simulink model "TosAeb"  (R2021a)
settings: Solver=FixedStepDiscrete  StartTime=0.0  StopTime=inf  FixedStep=0.01  RelTol=1e-3
undefined symbols (not defined in the file or in its folder): ACCModSts, AccSts1, AEB_ObjectMovingOrientation, AEB_ObjectMovingState, CN_BTCActive, CN_CurrentGearR, CN_CurrentGearR_DHT, CN_PTCActive, CN_VDCActive, CN_VSC_EspOff, CN_VSC_FCW_AEB_Error, CN_VSC_FCW_AEB_NoDispay, CN_VSC_FCW_AEB_PerfmDgdtn, CN_VSC_FCW_AEB_TempUnAvlbl, CN_VSC_VehStandstill, CNaccelAvoidMax, CNaccelAvoidMin, CNbrakePedalPress, CNcomfortStrLeftAccelMax, CNcomfortStrLeftAccelMin, CNcomfortStrRightAccelMax, CNcomfortStrRightAccelMin, CNDrvDoorOpen, CNDrvModeEco, CNDrvModeNorm, CNDrvModeOther, CNDrvModeSport, CNDrvSBRreminder, CNNull, CNobjInvalidID, CNPassDoorOpen, CNPassSBRreminder, CNPEBSAccelNegInf, CNPEBSAccelNull, CNPEBSTimeInf, CNPEBSTimeNull, CNSTOPMAX, CNSTOPNULL, CNsubjWidth, CNSwtOFF, CNSwtON, CNTTCMAX, CNTTCNULL, CNVehConfigAEBCycNone, CNVehConfigAEBNone, CNVehConfigAEBPedNone, CNVehConfigFCWNone, CNVehConfigJANone, CollisionSeverity, FALSE, FCWSnvty, JACycObjCalcInfoDefaultParams, JAPedObjCalcInfoDefaultParams, JAVehObjCalcInfoDefaultParams, latCycObjCalcInfoDefaultParams, latPedObjCalcInfoDefaultParams, longObjCalcInfoDefaultParams, ModSts, movingStatus, ObjectType ... (+1112)
libraries: simulink x3031, AEBSFunctionLib x50, Ta_Library x33, AEBSStateMachineLib x23, Mylibrary x12, simulink_extras x7, ObjSelect_lib x3, dspstat3 x2
(part 41 of 102)

== system TosAeb/FcwAebJa_Step_sys/ALG_AEBSystem/ALG_AEBController/AEB_FuncCondCalcApp/LongFunctionCondCalc/vehAEBpartSuppAborCondCalc/vehAEBpartAborCondCalc/vehAEBpartSituationRelAborCondCalc ==
  overlapRatio : Inport
  dxFrontBumper : Inport  Port=2
  probEscape : Inport  Port=3
  Compare To Constant3 : simulink/Logic and Bit Operations/Compare To Constant  relop=>  const=P_AEB_Func_vehAEBpartEscapeAborThres  OutDataTypeStr=boolean
  Compare To Constant6 : simulink/Logic and Bit Operations/Compare To Constant  relop===  const=FALSE  OutDataTypeStr=boolean
  Logical Operator1 : Logic  Operator=OR  AllPortsSameDT=off  OutDataTypeStr=boolean
  OverlapThesLookupTable : Lookup_n-D  NumberOfTableDimensions=1  BreakpointsForDimension1=P_AEB_Func_vehAEBpartOverlapAborThresX  BeginIndexSearchUsingPreviousIndexResult=on  Table=P_AEB_Func_vehAEBpartOverlapAborThresY  ExtrapMethod=Clip  InternalRulePriority=Speed  RndMeth=Simplest  InputSameDT=on
  Relational Operator : RelationalOperator  Operator=>  OutDataTypeStr=boolean  RndMeth=Simplest
  vehAEBpartSituationRelAborCondFulfilled : Outport
  wires:
  probEscape/1 -> Compare To Constant3/1
  dxFrontBumper/1 -> OverlapThesLookupTable/1
  OverlapThesLookupTable/1 -> Relational Operator/2
  Relational Operator/1 -> Compare To Constant6/1  "OverlapCondFulfilled"
  Compare To Constant3/1 -> Logical Operator1/2  "probEscapeFulfilled"
  overlapRatio/1 -> Relational Operator/1
  Logical Operator1/1 -> vehAEBpartSituationRelAborCondFulfilled/1
  Compare To Constant6/1 -> Logical Operator1/1  "OverlapCondFulfilled"

== system TosAeb/FcwAebJa_Step_sys/ALG_AEBSystem/ALG_AEBController/AEB_FuncCondCalcApp/LongFunctionCondCalc/vehAEBpartSuppAborCondCalc/vehAEBpartAborCondCalc/vehAEBpartVehRelAborCondCalc ==
  SteerWheelAngle : Inport
  SteerWheelSpd : Inport  Port=2
  AccrPedlPosnDiagc : Inport  Port=3
  vehStateCalcAEBpartAborFlg : Inport  Port=4
  DriverAborCondJudge : Subsystem
  vehPreWarningVehRelActiveCondJudge : Subsystem
  vehAEBpartVehRelAborCondFulfilled : Outport
  wires:
  vehStateCalcAEBpartAborFlg/1 -> vehPreWarningVehRelActiveCondJudge/2(vehStateCalcAEBpartAborFlg)
  AccrPedlPosnDiagc/1 -> DriverAborCondJudge/3(AccrPedlPosnDiagc)
  SteerWheelSpd/1 -> DriverAborCondJudge/2(SteerWheelSpd)
  SteerWheelAngle/1 -> DriverAborCondJudge/1(SteerWheelAngle)
  DriverAborCondJudge/1(driverAborCondFlg) -> vehPreWarningVehRelActiveCondJudge/1(driverAborCondFlg)
  vehPreWarningVehRelActiveCondJudge/1(vehAEBpartVehRelAborCondFulfilled) -> vehAEBpartVehRelAborCondFulfilled/1

== system TosAeb/FcwAebJa_Step_sys/ALG_AEBSystem/ALG_AEBController/AEB_FuncCondCalcApp/LongFunctionCondCalc/vehAEBpartSuppAborCondCalc/vehAEBpartSuppCondCalc/vehAEBpartObjRelActiveCondCalc ==
  ObjectMovingState : Inport
  ObjectTypeCameraConfirmed : Inport  Port=2
  ObjectType : Inport  Port=3
  subjCurvTraj : Inport  Port=4
  subjLatAccel : Inport  Port=5
  subjVel : Inport  Port=6
  vehConfigAEBCyc : Inport  Port=7
  vehConfigAEBPed : Inport  Port=8
  AEB_PedResp : Inport  Port=9
  ObjectMovingOrientation : Inport  Port=10
  ObjectID : Inport  Port=11
  ObjectExistProb : Inport  Port=12
  ObjectIsObstacleProb : Inport  Port=13
  dxVariance : Inport  Port=14
  dxFrontBumper : Inport  Port=15
  ObjFusQuality : Inport  Port=16
  AEBpartVruFuncConfig : Subsystem
  Compare To Constant1 : simulink/Logic and Bit Operations/Compare To Constant  relop===  const=AEB_ObjectMovingState.Moving  OutDataTypeStr=boolean
  Compare To Constant10 : simulink/Logic and Bit Operations/Compare To Constant  relop=<  const=P_AEB_Func_vehAEBpartDxActivationThres  OutDataTypeStr=boolean
  Compare To Constant11 : simulink/Logic and Bit Operations/Compare To Constant  relop===  const=TRUE  OutDataTypeStr=boolean
  Compare To Constant12 : simulink/Logic and Bit Operations/Compare To Constant  relop===  const=FALSE  OutDataTypeStr=boolean
  Compare To Constant2 : simulink/Logic and Bit Operations/Compare To Constant  relop===  const=AEB_ObjectMovingState.Stopped  OutDataTypeStr=boolean
  Compare To Constant3 : simulink/Logic and Bit Operations/Compare To Constant  relop===  const=AEB_ObjectMovingOrientation.SameDir  OutDataTypeStr=boolean
  Compare To Constant4 : simulink/Logic and Bit Operations/Compare To Constant  relop=~=  const=CNobjInvalidID  OutDataTypeStr=boolean
  Compare To Constant5 : simulink/Logic and Bit Operations/Compare To Constant  relop=>  const=P_AEB_Func_vehAEBpartObjObstacleProbActivationThres  OutDataTypeStr=boolean
  Compare To Constant6 : simulink/Logic and Bit Operations/Compare To Constant  relop=~=  const=CNobjInvalidID  OutDataTypeStr=boolean
  Compare To Constant7 : simulink/Logic and Bit Operations/Compare To Constant  relop===  const=TRUE  OutDataTypeStr=boolean
  Compare To Constant8 : simulink/Logic and Bit Operations/Compare To Constant  relop===  const=TRUE  OutDataTypeStr=boolean
  Compare To Constant9 : simulink/Logic and Bit Operations/Compare To Constant  relop=<  const=P_AEB_Func_vehAEBpartDxVarActivationThres  OutDataTypeStr=boolean
  Constant1 : Constant  Value=P_AEB_Func_vehAEBpartSubjVelActivationThresForPedCyc
  Constant2 : Constant  Value=P_AEB_Func_vehAEBpartSubjCurvTrajActivationThresForPedCyc
  Constant3 : Constant  Value=P_AEB_Func_vehAEBpartSubjLatAccelActivationThresForPedCyc
  Logical Operator : Logic  Operator=OR  AllPortsSameDT=off  OutDataTypeStr=boolean
  Logical Operator1 : Logic  AllPortsSameDT=off  OutDataTypeStr=boolean
  Logical Operator2 : Logic  AllPortsSameDT=off  OutDataTypeStr=boolean
  Logical Operator5 : Logic  Inputs=10  AllPortsSameDT=off  OutDataTypeStr=boolean
  PedCycObjCondSuppJudgeFlow : AEBSFunctionLib/PedCycObjCondSuppJudgeFlow  SystemSampleTime=-1  VariantActivationTime=update diagram  Latency=0  AutoFrameSizeCalculation=off
  Product : Product
  Relational Operator : RelationalOperator  Operator===  OutDataTypeStr=boolean  RndMeth=Simplest
  Unit Delay : UnitDelay  SampleTime=-1  HasFrameUpgradeWarning=on
  vehAEBpartObjRelActiveCondFulfilled : Outport
  wires:
  Compare To Constant11/1 -> Logical Operator5/4  "vehAEBpartVruFuncConfig"
  AEBpartVruFuncConfig/1(vehAEBpartVruFuncConfig) -> Compare To Constant11/1
  Compare To Constant7/1 -> Logical Operator5/10  "ObjFusQuality"
  ObjFusQuality/1 -> Compare To Constant7/1
  ObjectMovingState/1 -> Compare To Constant2/1, Compare To Constant1/1
  Compare To Constant8/1 -> Logical Operator5/2  "ObjFusionCamerainfoConfm"
  Constant3/1 -> PedCycObjCondSuppJudgeFlow/11
  Constant2/1 -> PedCycObjCondSuppJudgeFlow/10
  Constant1/1 -> PedCycObjCondSuppJudgeFlow/9
  AEB_PedResp/1 -> PedCycObjCondSuppJudgeFlow/8
  vehConfigAEBPed/1 -> PedCycObjCondSuppJudgeFlow/7
  vehConfigAEBCyc/1 -> PedCycObjCondSuppJudgeFlow/6
  subjVel/1 -> PedCycObjCondSuppJudgeFlow/5
  subjLatAccel/1 -> PedCycObjCondSuppJudgeFlow/4
  subjCurvTraj/1 -> PedCycObjCondSuppJudgeFlow/3
  ObjectType/1 -> PedCycObjCondSuppJudgeFlow/2, AEBpartVruFuncConfig/1(ObjectType)
  ObjectTypeCameraConfirmed/1 -> PedCycObjCondSuppJudgeFlow/1, Compare To Constant8/1
  Compare To Constant9/1 -> Logical Operator5/8  "dxRelVarCondFulfilled"
  Logical Operator1/1 -> Logical Operator5/6  "objIDCondFulfilled"
  Logical Operator/1 -> Logical Operator5/1  "objMovingStateCondFulfilled"
  Compare To Constant10/1 -> Logical Operator5/9  "dxRelCondFulfilled"
  PedCycObjCondSuppJudgeFlow/1 -> Compare To Constant12/1  "pedCycObjCondSuppFlg"
  Logical Operator2/1 -> Logical Operator5/7  "objQualityCondFulfilled"
  Compare To Constant3/1 -> Logical Operator5/5  "objMovingOrientationCondFulfilled"
  Logical Operator5/1 -> vehAEBpartObjRelActiveCondFulfilled/1  "vehAEBpartObjRelActiveCondFulfilled"
  Compare To Constant4/1 -> Logical Operator1/1
  Relational Operator/1 -> Logical Operator1/2
  dxVariance/1 -> Compare To Constant9/1
  dxFrontBumper/1 -> Compare To Constant10/1
  Product/1 -> Compare To Constant5/1
  Compare To Constant5/1 -> Logical Operator2/2
  Compare To Constant6/1 -> Logical Operator2/1
  ObjectIsObstacleProb/1 -> Product/2
  ObjectExistProb/1 -> Product/1
  Unit Delay/1 -> Relational Operator/2
  ObjectID/1 -> Compare To Constant4/1, Relational Operator/1, Unit Delay/1, Compare To Constant6/1
  ObjectMovingOrientation/1 -> Compare To Constant3/1
  Compare To Constant1/1 -> Logical Operator/1
  Compare To Constant2/1 -> Logical Operator/2
  Compare To Constant12/1 -> Logical Operator5/3  "pedCycReleventCondFulfilled"

== system TosAeb/FcwAebJa_Step_sys/ALG_AEBSystem/ALG_AEBController/AEB_FuncCondCalcApp/LongFunctionCondCalc/vehAEBpartSuppAborCondCalc/vehAEBpartSuppCondCalc/vehAEBpartSituationRelActiveCondCalc ==
  ObjectTypeCameraConfirmed : Inport
  dxFrontBumper : Inport  Port=2
  longCollisionSeverity : Inport  Port=3
  overlapRatio : Inport  Port=4
  LCK_Active : Inport  Port=5
  probEscape : Inport  Port=6
  subjCurvTraj : Inport  Port=7
  overlapThesCalc : Subsystem
  vehAEBpartSituationRelActiveCondCalc : Subsystem
  vehAEBpartSituationRelActiveCondFulfilled : Outport
  wires:
  LCK_Active/1 -> vehAEBpartSituationRelActiveCondCalc/4(LCK_Active)
  vehAEBpartSituationRelActiveCondCalc/1(vehAEBpartSituationRelActiveCondFulfilled) -> vehAEBpartSituationRelActiveCondFulfilled/1  "vehAEBpartSituationRelActiveCondFulfilled"
  subjCurvTraj/1 -> vehAEBpartSituationRelActiveCondCalc/6(subjCurvTraj)
  probEscape/1 -> vehAEBpartSituationRelActiveCondCalc/5(probEscape)
  overlapThesCalc/1(overlapThes) -> vehAEBpartSituationRelActiveCondCalc/3(OverlapThes)
  overlapRatio/1 -> vehAEBpartSituationRelActiveCondCalc/2(overlapRatio)
  longCollisionSeverity/1 -> vehAEBpartSituationRelActiveCondCalc/1(longCollisionSeverity)
  ObjectTypeCameraConfirmed/1 -> overlapThesCalc/1(ObjectTypeCameraConfirmed)
  dxFrontBumper/1 -> overlapThesCalc/2(dxFrontBumper)

== system TosAeb/FcwAebJa_Step_sys/ALG_AEBSystem/ALG_AEBController/AEB_FuncCondCalcApp/LongFunctionCondCalc/vehAEBpartSuppAborCondCalc/vehAEBpartSuppCondCalc/vehAEBpartSuppCondFinalJudge ==
  vehAEBpartObjRelActiveCondFulfilled : Inport
  vehAEBpartVehRelActiveCondFulfilled : Inport  Port=2
  vehAEBpartSituationRelActiveCondFulfilled : Inport  Port=3
  Compare To Constant : simulink/Logic and Bit Operations/Compare To Constant  relop===  const=FALSE  OutDataTypeStr=boolean
  Compare To Constant1 : simulink/Logic and Bit Operations/Compare To Constant  relop===  const=FALSE  OutDataTypeStr=boolean
  Compare To Constant2 : simulink/Logic and Bit Operations/Compare To Constant  relop===  const=FALSE  OutDataTypeStr=boolean
  Logical Operator : Logic  Operator=OR  Inputs=3  AllPortsSameDT=off  OutDataTypeStr=boolean
  vehAEBpartSuppCondFulfilled : Outport
  wires:
  Compare To Constant2/1 -> Logical Operator/3
  Compare To Constant1/1 -> Logical Operator/2
  Compare To Constant/1 -> Logical Operator/1
  Logical Operator/1 -> vehAEBpartSuppCondFulfilled/1  "vehAEBpartSuppCondFulfilled"
  vehAEBpartSituationRelActiveCondFulfilled/1 -> Compare To Constant2/1  "vehAEBpartSituationRelActiveCondFulfilled"
  vehAEBpartObjRelActiveCondFulfilled/1 -> Compare To Constant/1  "vehAEBpartObjRelActiveCondFulfilled"
  vehAEBpartVehRelActiveCondFulfilled/1 -> Compare To Constant1/1  "vehAEBpartVehRelActiveCondFulfilled"

== system TosAeb/FcwAebJa_Step_sys/ALG_AEBSystem/ALG_AEBController/AEB_FuncCondCalcApp/LongFunctionCondCalc/vehAEBpartSuppAborCondCalc/vehAEBpartSuppCondCalc/vehAEBpartVehRelActiveCondCalc ==
  subjVel : Inport
  SteerWheelAngle : Inport  Port=2
  SteerWheelSpd : Inport  Port=3
  AccrPedlPosnDiagc : Inport  Port=4
  VehConfigIbooster : Inport  Port=5
  ESPmasterCylindBrakePress : Inport  Port=6
  BrakePedalStatus_G : Inport  Port=7
  vehStateCalcAEBpartSuppFlg : Inport  Port=8
  IB_BrkPedalAppld : Inport  Port=9
  DriverCondJudge : Subsystem
  vehAEBpartVehRelActiveCondJudge : Subsystem
  vehAEBpartVehRelActiveCondFulfilled : Outport
  wires:
  VehConfigIbooster/1 -> DriverCondJudge/4(VehConfigIbooster)
  IB_BrkPedalAppld/1 -> DriverCondJudge/7(IB_BrkPedalAppld)
  vehAEBpartVehRelActiveCondJudge/1(vehAEBpartVehRelActiveCondFulfilled) -> vehAEBpartVehRelActiveCondFulfilled/1  "vehAEBpartVehRelActiveCondFulfilled"
  vehStateCalcAEBpartSuppFlg/1 -> vehAEBpartVehRelActiveCondJudge/3(vehStateCalcAEBpartSuppFlg)
  subjVel/1 -> vehAEBpartVehRelActiveCondJudge/1(subjVel)
  DriverCondJudge/1(driverSuppCondFlg) -> vehAEBpartVehRelActiveCondJudge/2(driverSuppCondFlg)
  AccrPedlPosnDiagc/1 -> DriverCondJudge/3(AccrPedlPosnDiagc)
  ESPmasterCylindBrakePress/1 -> DriverCondJudge/5(ESPmasterCylindBrakePress)
  SteerWheelSpd/1 -> DriverCondJudge/2(SteerWheelSpd)
  SteerWheelAngle/1 -> DriverCondJudge/1(SteerWheelAngle)
  BrakePedalStatus_G/1 -> DriverCondJudge/6(BrakePedalStatus_G)

== system TosAeb/FcwAebJa_Step_sys/ALG_AEBSystem/ALG_AEBController/AEB_FuncCondCalcApp/LongFunctionCondCalc/vehAcuteWarningSuppAborCondFulfilled/vehAcuteWarningAborCondCalc/vehAcuteWarningAborCondFinalJudge ==
  vehAcuteWarningObjRelAborCondFulfilled : Inport
  vehAcuteWarningVehRelAborCondFulfilled : Inport  Port=2
  vehAcuteWarningSituationRelAborCondFulfilled : Inport  Port=3
  Compare To Constant1 : simulink/Logic and Bit Operations/Compare To Constant  relop===  const=TRUE  OutDataTypeStr=boolean
  Compare To Constant2 : simulink/Logic and Bit Operations/Compare To Constant  relop===  const=TRUE  OutDataTypeStr=boolean
  Compare To Constant3 : simulink/Logic and Bit Operations/Compare To Constant  relop===  const=TRUE  OutDataTypeStr=boolean
  Logical Operator : Logic  Operator=OR  Inputs=3  AllPortsSameDT=off  OutDataTypeStr=boolean
  vehAcuteWarningAborCond : Outport
  wires:
  Compare To Constant3/1 -> Logical Operator/3
  Compare To Constant2/1 -> Logical Operator/2
  Compare To Constant1/1 -> Logical Operator/1
  vehAcuteWarningVehRelAborCondFulfilled/1 -> Compare To Constant2/1
  vehAcuteWarningObjRelAborCondFulfilled/1 -> Compare To Constant1/1
  vehAcuteWarningSituationRelAborCondFulfilled/1 -> Compare To Constant3/1
  Logical Operator/1 -> vehAcuteWarningAborCond/1  "vehAcuteWarningAborCond"

== system TosAeb/FcwAebJa_Step_sys/ALG_AEBSystem/ALG_AEBController/AEB_FuncCondCalcApp/LongFunctionCondCalc/vehAcuteWarningSuppAborCondFulfilled/vehAcuteWarningAborCondCalc/vehAcuteWarningObjRelAborCondCalc ==
  ObjectID : Inport
  Compare To Constant4 : simulink/Logic and Bit Operations/Compare To Constant  relop===  const=CNobjInvalidID  OutDataTypeStr=boolean
  Logical Operator1 : Logic  Operator=OR  AllPortsSameDT=off  OutDataTypeStr=boolean
  Relational Operator : RelationalOperator  Operator=~=  OutDataTypeStr=boolean  RndMeth=Simplest
  Unit Delay : UnitDelay  SampleTime=-1  HasFrameUpgradeWarning=on
  vehAcuteWarningObjRelAborCondFulfilled : Outport
  wires:
  Logical Operator1/1 -> vehAcuteWarningObjRelAborCondFulfilled/1  "vehAcuteWarningObjRelAborCondFulfilled"
  Compare To Constant4/1 -> Logical Operator1/1
  Relational Operator/1 -> Logical Operator1/2
  Unit Delay/1 -> Relational Operator/2
  ObjectID/1 -> Compare To Constant4/1, Relational Operator/1, Unit Delay/1

== system TosAeb/FcwAebJa_Step_sys/ALG_AEBSystem/ALG_AEBController/AEB_FuncCondCalcApp/LongFunctionCondCalc/vehAcuteWarningSuppAborCondFulfilled/vehAcuteWarningAborCondCalc/vehAcuteWarningSituationRelAborCondCalc ==
  overlapRatio : Inport
  dxFrontBumper : Inport  Port=2
  probEscape : Inport  Port=3
  Compare To Constant3 : simulink/Logic and Bit Operations/Compare To Constant  relop=>  const=P_AEB_Func_vehAcuteWarningEscapeAborThres  OutDataTypeStr=boolean
  Compare To Constant6 : simulink/Logic and Bit Operations/Compare To Constant  relop===  const=FALSE  OutDataTypeStr=boolean
  Logical Operator1 : Logic  AllPortsSameDT=off  OutDataTypeStr=boolean
  OverlapThesLookupTable : Lookup_n-D  NumberOfTableDimensions=1  BreakpointsForDimension1=P_AEB_Func_vehAcuteWarningOverlapAborThresX  BeginIndexSearchUsingPreviousIndexResult=on  Table=P_AEB_Func_vehAcuteWarningOverlapAborThresY  ExtrapMethod=Clip  InternalRulePriority=Speed  RndMeth=Simplest  InputSameDT=on
  Relational Operator : RelationalOperator  Operator=>  OutDataTypeStr=boolean  RndMeth=Simplest
  vehAcuteWarningSituationRelAborCondFulfilled : Outport
  wires:
  Compare To Constant6/1 -> Logical Operator1/1
  Logical Operator1/1 -> vehAcuteWarningSituationRelAborCondFulfilled/1  "vehAcuteWarningSituationRelABorCondFulfilled"
  overlapRatio/1 -> Relational Operator/1
  Compare To Constant3/1 -> Logical Operator1/2  "probEscapeFulfilled"
  Relational Operator/1 -> Compare To Constant6/1  "OverlapCondFulfilled"
  OverlapThesLookupTable/1 -> Relational Operator/2
  dxFrontBumper/1 -> OverlapThesLookupTable/1
  probEscape/1 -> Compare To Constant3/1

== system TosAeb/FcwAebJa_Step_sys/ALG_AEBSystem/ALG_AEBController/AEB_FuncCondCalcApp/LongFunctionCondCalc/vehAcuteWarningSuppAborCondFulfilled/vehAcuteWarningAborCondCalc/vehAcuteWarningVehRelAborCondCalc ==
  SteerWheelAngle : Inport
  SteerWheelSpd : Inport  Port=2
  ESPmasterCylindBrakePress : Inport  Port=3
  vehStateCalcAcuteWarningAborFlg : Inport  Port=4
  DriverAborCondJudge : Subsystem
  vehPreWarningVehRelActiveCondJudge : Subsystem
  vehAcuteWarningVehRelAborCondFulfilled : Outport
  wires:
  vehPreWarningVehRelActiveCondJudge/1(vehAcuteWarningVehRelAborCondFulfilled) -> vehAcuteWarningVehRelAborCondFulfilled/1  "vehAcuteWarningVehRelAborCondFulfilled"
  vehStateCalcAcuteWarningAborFlg/1 -> vehPreWarningVehRelActiveCondJudge/2(vehStateCalcAcuteWarningAborFlg)
  DriverAborCondJudge/1(driverAborCondFlg) -> vehPreWarningVehRelActiveCondJudge/1(driverAborCondFlg)
  ESPmasterCylindBrakePress/1 -> DriverAborCondJudge/3(AccrPedlPosnDiagc)
  SteerWheelSpd/1 -> DriverAborCondJudge/2(SteerWheelSpd)
  SteerWheelAngle/1 -> DriverAborCondJudge/1(SteerWheelAngle)

== system TosAeb/FcwAebJa_Step_sys/ALG_AEBSystem/ALG_AEBController/AEB_FuncCondCalcApp/LongFunctionCondCalc/vehAcuteWarningSuppAborCondFulfilled/vehAcuteWarningSuppCondCalc/vehAcuteWarningObjRelActiveCondCalc ==
  ObjectMovingState : Inport
  dz : Inport  Port=2
  ObjectTypeCameraConfirmed : Inport  Port=3
  ObjectType : Inport  Port=4
  subjCurvTraj : Inport  Port=5
  subjLatAccel : Inport  Port=6
  subjVel : Inport  Port=7
  vehConfigAEBCyc : Inport  Port=8
  vehConfigAEBPed : Inport  Port=9
  AEB_PedResp : Inport  Port=10
  ObjectMovingOrientation : Inport  Port=11
  ObjectID : Inport  Port=12
  ObjectExistProb : Inport  Port=13
  ObjectIsObstacleProb : Inport  Port=14
  dxVariance : Inport  Port=15
  dxFrontBumper : Inport  Port=16
  ObjFusQuality : Inport  Port=17
  Compare To Constant : simulink/Logic and Bit Operations/Compare To Constant  relop===  const=AEB_ObjectMovingState.Still  OutDataTypeStr=boolean
  Compare To Constant1 : simulink/Logic and Bit Operations/Compare To Constant  relop===  const=AEB_ObjectMovingState.Moving  OutDataTypeStr=boolean
  Compare To Constant10 : simulink/Logic and Bit Operations/Compare To Constant  relop=<  const=P_AEB_Func_vehAcuteWarningDxActivationThres  OutDataTypeStr=boolean
  Compare To Constant11 : simulink/Logic and Bit Operations/Compare To Constant  relop===  const=TRUE  OutDataTypeStr=boolean
  Compare To Constant12 : simulink/Logic and Bit Operations/Compare To Constant  relop===  const=FALSE  OutDataTypeStr=boolean
  Compare To Constant14 : simulink/Logic and Bit Operations/Compare To Constant  relop===  const=AEB_ObjectMovingState.Still  OutDataTypeStr=boolean
  Compare To Constant2 : simulink/Logic and Bit Operations/Compare To Constant  relop===  const=AEB_ObjectMovingState.Stopped  OutDataTypeStr=boolean
  Compare To Constant3 : simulink/Logic and Bit Operations/Compare To Constant  relop===  const=AEB_ObjectMovingOrientation.SameDir  OutDataTypeStr=boolean
  Compare To Constant4 : simulink/Logic and Bit Operations/Compare To Constant  relop=~=  const=CNobjInvalidID  OutDataTypeStr=boolean
  Compare To Constant5 : simulink/Logic and Bit Operations/Compare To Constant  relop=>  const=P_AEB_Func_vehAcuteWarningObjObstacleProbActivationThres  OutDataTypeStr=boolean
  Compare To Constant6 : simulink/Logic and Bit Operations/Compare To Constant  relop=~=  const=CNobjInvalidID  OutDataTypeStr=boolean
  Compare To Constant7 : simulink/Logic and Bit Operations/Compare To Constant  relop=<  const=P_AEB_Func_vehAcuteWarningObjHeightActivationThres  OutDataTypeStr=boolean
  Compare To Constant8 : simulink/Logic and Bit Operations/Compare To Constant  relop===  const=TRUE  OutDataTypeStr=boolean
  Compare To Constant9 : simulink/Logic and Bit Operations/Compare To Constant  relop=<  const=P_AEB_Func_vehAcuteWarningDxVarActivationThres  OutDataTypeStr=boolean
  Constant : Constant  Value=TRUE
  Constant1 : Constant  Value=P_AEB_Func_vehAcuteWarningSubjVelActivationThresForPedCyc
  Constant2 : Constant  Value=P_AEB_Func_vehAcuteWarningSubjCurvTrajActivationThresForPedCyc
  Constant3 : Constant  Value=P_AEB_Func_vehAcuteWarningSubjLatAccelActivationThresForPedCyc
  Logical Operator : Logic  Operator=OR  Inputs=3  AllPortsSameDT=off  OutDataTypeStr=boolean
  Logical Operator1 : Logic  AllPortsSameDT=off  OutDataTypeStr=boolean
  Logical Operator2 : Logic  AllPortsSameDT=off  OutDataTypeStr=boolean
  Logical Operator5 : Logic  Inputs=10  AllPortsSameDT=off  OutDataTypeStr=boolean
  PedCycObjCondSuppJudgeFlow : AEBSFunctionLib/PedCycObjCondSuppJudgeFlow  SystemSampleTime=-1  VariantActivationTime=update diagram  Latency=0  AutoFrameSizeCalculation=off
  Product : Product
  Relational Operator : RelationalOperator  Operator===  OutDataTypeStr=boolean  RndMeth=Simplest
  Switch : Switch  Criteria=u2 ~= 0
  Unit Delay : UnitDelay  SampleTime=-1  HasFrameUpgradeWarning=on
  vehAcuteWarningObjRelActiveCondFulfilled : Outport
  wires:
  Compare To Constant11/1 -> Logical Operator5/10  "ObjFusQuality"
  ObjFusQuality/1 -> Compare To Constant11/1
  Compare To Constant8/1 -> Logical Operator5/3  "objCameraConfmFullfilled"
  Constant3/1 -> PedCycObjCondSuppJudgeFlow/11
  Constant2/1 -> PedCycObjCondSuppJudgeFlow/10
  Constant1/1 -> PedCycObjCondSuppJudgeFlow/9
  subjCurvTraj/1 -> PedCycObjCondSuppJudgeFlow/3
  subjLatAccel/1 -> PedCycObjCondSuppJudgeFlow/4
  subjVel/1 -> PedCycObjCondSuppJudgeFlow/5
  vehConfigAEBCyc/1 -> PedCycObjCondSuppJudgeFlow/6
  vehConfigAEBPed/1 -> PedCycObjCondSuppJudgeFlow/7
  AEB_PedResp/1 -> PedCycObjCondSuppJudgeFlow/8
  ObjectType/1 -> PedCycObjCondSuppJudgeFlow/2
  ObjectTypeCameraConfirmed/1 -> PedCycObjCondSuppJudgeFlow/1, Compare To Constant8/1
  Constant/1 -> Switch/3
  Compare To Constant7/1 -> Switch/1
  ObjectMovingState/1 -> Compare To Constant14/1, Compare To Constant/1, Compare To Constant2/1, Compare To Constant1/1
  Compare To Constant14/1 -> Switch/2
  Compare To Constant12/1 -> Logical Operator5/4  "pedCycReleventCondFulfilled"
  Switch/1 -> Logical Operator5/2  "objHeightInfoFulfilled"
  Compare To Constant2/1 -> Logical Operator/2
  Compare To Constant1/1 -> Logical Operator/1
  ObjectMovingOrientation/1 -> Compare To Constant3/1
  ObjectID/1 -> Compare To Constant6/1, Unit Delay/1, Relational Operator/1, Compare To Constant4/1
  Unit Delay/1 -> Relational Operator/2
  ObjectExistProb/1 -> Product/1
  ObjectIsObstacleProb/1 -> Product/2
  Compare To Constant6/1 -> Logical Operator2/1
  Compare To Constant5/1 -> Logical Operator2/2
  Product/1 -> Compare To Constant5/1
  dxFrontBumper/1 -> Compare To Constant10/1
  dxVariance/1 -> Compare To Constant9/1
  dz/1 -> Compare To Constant7/1
  Compare To Constant/1 -> Logical Operator/3
  Relational Operator/1 -> Logical Operator1/2
  Compare To Constant4/1 -> Logical Operator1/1
  Logical Operator5/1 -> vehAcuteWarningObjRelActiveCondFulfilled/1  "vehAcuteWarningObjRelActiveCondFulfilled"
  Compare To Constant3/1 -> Logical Operator5/5  "objMovingOrientationCondFulfilled"
  Logical Operator2/1 -> Logical Operator5/7  "objQualityCondFulfilled"
  PedCycObjCondSuppJudgeFlow/1 -> Compare To Constant12/1  "pedCycObjCondSuppFlg"
  Compare To Constant10/1 -> Logical Operator5/9  "dxRelCondFulfilled"
  Logical Operator/1 -> Logical Operator5/1  "objMovingStateCondFulfilled"
  Logical Operator1/1 -> Logical Operator5/6  "objIDCondFulfilled"
  Compare To Constant9/1 -> Logical Operator5/8  "dxRelVarCondFulfilled"

== system TosAeb/FcwAebJa_Step_sys/ALG_AEBSystem/ALG_AEBController/AEB_FuncCondCalcApp/LongFunctionCondCalc/vehAcuteWarningSuppAborCondFulfilled/vehAcuteWarningSuppCondCalc/vehAcuteWarningSituationRelActiveCondCalc ==
  longCollisionSeverity : Inport
  overlapRatio : Inport  Port=2
  dxFrontBumper : Inport  Port=3
  probEscape : Inport  Port=4
  subjCurvTraj : Inport  Port=5
  Compare To Constant1 : simulink/Logic and Bit Operations/Compare To Constant  relop===  const=CollisionSeverity.Medium  OutDataTypeStr=boolean
  Compare To Constant2 : simulink/Logic and Bit Operations/Compare To Constant  relop===  const=CollisionSeverity.High  OutDataTypeStr=boolean
  Compare To Constant3 : simulink/Logic and Bit Operations/Compare To Constant  relop=>  const=P_AEB_Func_vehAcuteWarningEscapeActivationThres  OutDataTypeStr=boolean
  Compare To Constant4 : simulink/Logic and Bit Operations/Compare To Constant  relop=<  const=P_AEB_Func_vehAcuteWarningSubjCurvTrajActivationThres  OutDataTypeStr=boolean
  Compare To Constant5 : simulink/Logic and Bit Operations/Compare To Constant  relop===  const=FALSE  OutDataTypeStr=boolean
  Logical Operator : Logic  Operator=OR  AllPortsSameDT=off  OutDataTypeStr=boolean
  Logical Operator1 : Logic  Inputs=4  AllPortsSameDT=off  OutDataTypeStr=boolean
  OverlapThesLookupTable : Lookup_n-D  NumberOfTableDimensions=1  BreakpointsForDimension1=P_AEB_Func_vehAcuteWarningOverlapActivationThresX  BeginIndexSearchUsingPreviousIndexResult=on  Table=P_AEB_Func_vehAcuteWarningOverlapActivationThresY  ExtrapMethod=Clip  InternalRulePriority=Speed  RndMeth=Simplest  InputSameDT=on
  Relational Operator : RelationalOperator  Operator=>  OutDataTypeStr=boolean  RndMeth=Simplest
  vehAcuteWarningSituationRelActiveCondFulfilled : Outport
  wires:
  longCollisionSeverity/1 -> Compare To Constant1/1, Compare To Constant2/1
  Compare To Constant1/1 -> Logical Operator/1
  Compare To Constant2/1 -> Logical Operator/2
  dxFrontBumper/1 -> OverlapThesLookupTable/1
  OverlapThesLookupTable/1 -> Relational Operator/2
  overlapRatio/1 -> Relational Operator/1
  probEscape/1 -> Compare To Constant3/1
  subjCurvTraj/1 -> Compare To Constant4/1
  Compare To Constant3/1 -> Compare To Constant5/1  "probEscapeFulfilled"
  Compare To Constant5/1 -> Logical Operator1/3  "probEscapeFulfilled"
  Relational Operator/1 -> Logical Operator1/2  "OverlapCondFulfilled"
  Compare To Constant4/1 -> Logical Operator1/4  "latStableCondFulfilled"
  Logical Operator/1 -> Logical Operator1/1  "collisionLvlCondFulfilled"
  Logical Operator1/1 -> vehAcuteWarningSituationRelActiveCondFulfilled/1

== system TosAeb/FcwAebJa_Step_sys/ALG_AEBSystem/ALG_AEBController/AEB_FuncCondCalcApp/LongFunctionCondCalc/vehAcuteWarningSuppAborCondFulfilled/vehAcuteWarningSuppCondCalc/vehAcuteWarningSuppCondFinalJudge ==
  vehAcuteWarningObjRelActiveCondFulfilled : Inport
  vehAcuteWarningVehRelActiveCondFulfilled : Inport  Port=2
  vehAcuteWarningSituationRelActiveCondFulfilled : Inport  Port=3
  Compare To Constant1 : simulink/Logic and Bit Operations/Compare To Constant  relop===  const=FALSE  OutDataTypeStr=boolean
  Compare To Constant2 : simulink/Logic and Bit Operations/Compare To Constant  relop===  const=FALSE  OutDataTypeStr=boolean
  Compare To Constant5 : simulink/Logic and Bit Operations/Compare To Constant  relop===  const=FALSE  OutDataTypeStr=boolean
  Logical Operator : Logic  Operator=OR  Inputs=3  AllPortsSameDT=off  OutDataTypeStr=boolean
  vehAcuteWarningSuppCond : Outport
  wires:
  Compare To Constant2/1 -> Logical Operator/3
  Compare To Constant1/1 -> Logical Operator/2
  Compare To Constant5/1 -> Logical Operator/1
  vehAcuteWarningVehRelActiveCondFulfilled/1 -> Compare To Constant1/1
  vehAcuteWarningObjRelActiveCondFulfilled/1 -> Compare To Constant5/1
  vehAcuteWarningSituationRelActiveCondFulfilled/1 -> Compare To Constant2/1
  Logical Operator/1 -> vehAcuteWarningSuppCond/1  "vehAcuteWarningSuppCond"

== system TosAeb/FcwAebJa_Step_sys/ALG_AEBSystem/ALG_AEBController/AEB_FuncCondCalcApp/LongFunctionCondCalc/vehAcuteWarningSuppAborCondFulfilled/vehAcuteWarningSuppCondCalc/vehAcuteWarningVehRelActiveCondCalc ==
  subjVel : Inport
  ObjectMovingState : Inport  Port=2
  SteerWheelAngle : Inport  Port=3
  SteerWheelSpd : Inport  Port=4
  AccrPedlPosnDiagc : Inport  Port=5
  VehConfigIbooster : Inport  Port=6
  ESPmasterCylindBrakePress : Inport  Port=7
  BrakePedalStatus_G : Inport  Port=8
  IB_BrkPedalAppld : Inport  Port=9
  vehStateCalcPreWarningSuppFlg : Inport  Port=10
  subjLongAccel : Inport  Port=11
  BrakeNeedCondJudge : Subsystem
  DriverCondJudge : Subsystem
  subjVelWork : Subsystem
  vehAcuteWarningVehRelActiveCondJudge : Subsystem
  vehAcuteWarningVehRelActiveCondFulfilled : Outport
  wires:
  VehConfigIbooster/1 -> DriverCondJudge/3(VehConfigIbooster)
  IB_BrkPedalAppld/1 -> DriverCondJudge/6(IB_BrkPedalAppld)
  BrakeNeedCondJudge/1(brakeNeedCondFulfilled) -> vehAcuteWarningVehRelActiveCondJudge/5(brakeNeedCondFulfilled)
  BrakePedalStatus_G/1 -> BrakeNeedCondJudge/1(BrakePedalStatus_G), DriverCondJudge/7(BrakePedalStatus_G)
  subjLongAccel/1 -> BrakeNeedCondJudge/2(subjLongAccel)
  ObjectMovingState/1 -> subjVelWork/1(ObjectMovingState)
  SteerWheelAngle/1 -> DriverCondJudge/1(SteerWheelAngle)
  SteerWheelSpd/1 -> DriverCondJudge/2(SteerWheelSpd)
  ESPmasterCylindBrakePress/1 -> DriverCondJudge/5(ESPmasterCylindBrakePress)
  AccrPedlPosnDiagc/1 -> DriverCondJudge/4(AccrPedlPosnDiagc)
  subjVelWork/1(subjVelWorkMax) -> vehAcuteWarningVehRelActiveCondJudge/2(subjVelWorkMax)
  subjVelWork/2(subjVelWorkMin) -> vehAcuteWarningVehRelActiveCondJudge/3(subjVelWorkMin)
  DriverCondJudge/1(driverSuppCondFlg) -> vehAcuteWarningVehRelActiveCondJudge/4(driverSuppCondFlg)
  subjVel/1 -> vehAcuteWarningVehRelActiveCondJudge/1(subjVel)
  vehStateCalcPreWarningSuppFlg/1 -> vehAcuteWarningVehRelActiveCondJudge/6(vehStateCalcPreWarningSuppFlg)
  vehAcuteWarningVehRelActiveCondJudge/1(vehAcuteWarningVehRelActiveCondFulfilled) -> vehAcuteWarningVehRelActiveCondFulfilled/1  "vehAcuteWarningVehRelActiveCondFulfilled"

== system TosAeb/FcwAebJa_Step_sys/ALG_AEBSystem/ALG_AEBController/AEB_FuncCondCalcApp/LongFunctionCondCalc/vehEBASuppAborCondCalc/vehEBAAborCondCalc/vehEBAAborCondFinalJudge ==
  vehEBAObjRelAborCondFulfilled : Inport
  vehEBAVehRelAborCondFulfilled : Inport  Port=2
  vehEBASituationRelAborCondFulfilled : Inport  Port=3
  Compare To Constant : simulink/Logic and Bit Operations/Compare To Constant  relop===  const=TRUE  OutDataTypeStr=boolean
  Compare To Constant1 : simulink/Logic and Bit Operations/Compare To Constant  relop===  const=TRUE  OutDataTypeStr=boolean
  Compare To Constant2 : simulink/Logic and Bit Operations/Compare To Constant  relop===  const=TRUE  OutDataTypeStr=boolean
  Logical Operator : Logic  Operator=OR  Inputs=3  AllPortsSameDT=off  OutDataTypeStr=boolean
  vehEBAAborCondNoStandstillFulfilled : Outport
  vehEBAAborCondStandstillFulfilled : Outport  Port=2
  wires:
  Compare To Constant2/1 -> Logical Operator/2
  Compare To Constant1/1 -> Logical Operator/3, vehEBAAborCondStandstillFulfilled/1
  Compare To Constant/1 -> Logical Operator/1
  Logical Operator/1 -> vehEBAAborCondNoStandstillFulfilled/1
  vehEBASituationRelAborCondFulfilled/1 -> Compare To Constant2/1
  vehEBAObjRelAborCondFulfilled/1 -> Compare To Constant/1
  vehEBAVehRelAborCondFulfilled/1 -> Compare To Constant1/1

== system TosAeb/FcwAebJa_Step_sys/ALG_AEBSystem/ALG_AEBController/AEB_FuncCondCalcApp/LongFunctionCondCalc/vehEBASuppAborCondCalc/vehEBAAborCondCalc/vehEBAObjRelAborCondCalc ==
  ObjectID : Inport
  Compare To Constant4 : simulink/Logic and Bit Operations/Compare To Constant  relop===  const=CNobjInvalidID  OutDataTypeStr=boolean
  Logical Operator1 : Logic  Operator=OR  AllPortsSameDT=off  OutDataTypeStr=boolean
  Relational Operator : RelationalOperator  Operator=~=  OutDataTypeStr=boolean  RndMeth=Simplest
  Unit Delay : UnitDelay  SampleTime=-1  HasFrameUpgradeWarning=on
  vehEBAObjRelAborCondFulfilled : Outport
  wires:
  ObjectID/1 -> Unit Delay/1, Relational Operator/1, Compare To Constant4/1
  Unit Delay/1 -> Relational Operator/2
  Relational Operator/1 -> Logical Operator1/2
  Compare To Constant4/1 -> Logical Operator1/1
  Logical Operator1/1 -> vehEBAObjRelAborCondFulfilled/1  "ObjIDAborCondFulfilled"

== system TosAeb/FcwAebJa_Step_sys/ALG_AEBSystem/ALG_AEBController/AEB_FuncCondCalcApp/LongFunctionCondCalc/vehEBASuppAborCondCalc/vehEBAAborCondCalc/vehEBASituationRelAborCondCalc ==
  Constant : Constant  Value=FALSE
  vehEBASituationRelAborCondFulfilled : Outport
  wires:
  Constant/1 -> vehEBASituationRelAborCondFulfilled/1

== system TosAeb/FcwAebJa_Step_sys/ALG_AEBSystem/ALG_AEBController/AEB_FuncCondCalcApp/LongFunctionCondCalc/vehEBASuppAborCondCalc/vehEBAAborCondCalc/vehEBAVehRelAborCondCalc ==
  BrakePedalStatus_G : Inport
  ESPmasterCylindBrakePress : Inport  Port=2
  SteerWheelAngle : Inport  Port=3
  SteerWheelSpd : Inport  Port=4
  AccrPedlPosnDiagc : Inport  Port=5
  vehStateCalcEBAAborFlg : Inport  Port=6
  BrkAborCondJudge : Subsystem
  DriverAborCondJudge : Subsystem
  vehPreWarningVehRelActiveCondJudge : Subsystem
  vehEBAVehRelAborCondFulfilled : Outport
  wires:
  AccrPedlPosnDiagc/1 -> DriverAborCondJudge/3(AccrPedlPosnDiagc)
  SteerWheelSpd/1 -> DriverAborCondJudge/2(SteerWheelSpd)
  SteerWheelAngle/1 -> DriverAborCondJudge/1(SteerWheelAngle)
  ESPmasterCylindBrakePress/1 -> BrkAborCondJudge/2(ESPmasterCylindBrakePress)
  BrakePedalStatus_G/1 -> BrkAborCondJudge/1(BrakePedalStatus_G)
  BrkAborCondJudge/1(BrkAborCondFlg) -> vehPreWarningVehRelActiveCondJudge/1(BrkAborCondFlg)
  DriverAborCondJudge/1(driverAborCondFlg) -> vehPreWarningVehRelActiveCondJudge/2(driverAborCondFlg)
  vehStateCalcEBAAborFlg/1 -> vehPreWarningVehRelActiveCondJudge/3(vehStateCalcEBAAborFlg)
  vehPreWarningVehRelActiveCondJudge/1(vehEBAVehRelAborCondFulfilled) -> vehEBAVehRelAborCondFulfilled/1

== system TosAeb/FcwAebJa_Step_sys/ALG_AEBSystem/ALG_AEBController/AEB_FuncCondCalcApp/LongFunctionCondCalc/vehEBASuppAborCondCalc/vehEBASuppCondCalc/vehEBAObjRelActiveCondCalc ==
  note: add the condition which juge whether the stand obj is fusion
  ObjectMovingState : Inport
  dxFrontBumper : Inport  Port=2
  ObjectTypeCameraConfirmed : Inport  Port=3
  ObjectType : Inport  Port=4
  subjCurvTraj : Inport  Port=5
  subjLatAccel : Inport  Port=6
  subjVel : Inport  Port=7
  vehConfigAEBCyc : Inport  Port=8
  vehConfigAEBPed : Inport  Port=9
  AEB_PedResp : Inport  Port=10
  ObjectMovingOrientation : Inport  Port=11
  ObjectID : Inport  Port=12
  ObjectExistProb : Inport  Port=13
  ObjectIsObstacleProb : Inport  Port=14
  dxVariance : Inport  Port=15
  ObjFusQuality : Inport  Port=16
  Compare To Constant : simulink/Logic and Bit Operations/Compare To Constant  relop===  const=AEB_ObjectMovingState.Still  OutDataTypeStr=boolean
  Compare To Constant1 : simulink/Logic and Bit Operations/Compare To Constant  relop===  const=AEB_ObjectMovingState.Moving  OutDataTypeStr=boolean
  Compare To Constant10 : simulink/Logic and Bit Operations/Compare To Constant  relop===  const=TRUE  OutDataTypeStr=boolean
  Compare To Constant12 : simulink/Logic and Bit Operations/Compare To Constant  relop===  const=FALSE  OutDataTypeStr=boolean
  Compare To Constant2 : simulink/Logic and Bit Operations/Compare To Constant  relop===  const=AEB_ObjectMovingState.Stopped  OutDataTypeStr=boolean
  Compare To Constant3 : simulink/Logic and Bit Operations/Compare To Constant  relop===  const=AEB_ObjectMovingOrientation.SameDir  OutDataTypeStr=boolean
  Compare To Constant4 : simulink/Logic and Bit Operations/Compare To Constant  relop=~=  const=CNobjInvalidID  OutDataTypeStr=boolean
  Compare To Constant5 : simulink/Logic and Bit Operations/Compare To Constant  relop=>  const=P_AEB_Func_vehEBAObjObstacleProbActivationThres  OutDataTypeStr=boolean
  Compare To Constant6 : simulink/Logic and Bit Operations/Compare To Constant  relop=~=  const=CNobjInvalidID  OutDataTypeStr=boolean
  Compare To Constant7 : simulink/Logic and Bit Operations/Compare To Constant  relop=<  const=P_AEB_Func_vehEBADxActivationThres  OutDataTypeStr=boolean
  Compare To Constant8 : simulink/Logic and Bit Operations/Compare To Constant  relop===  const=TRUE  OutDataTypeStr=boolean
  Compare To Constant9 : simulink/Logic and Bit Operations/Compare To Constant  relop=<  const=P_AEB_Func_vehEBADxVarActivationThres  OutDataTypeStr=boolean
  Constant3 : Constant  Value=P_AEB_Func_vehEBASubjVelActivationThresForPedCyc
  Constant4 : Constant  Value=P_AEB_Func_vehEBASubjCurvTrajActivationThresForPedCyc
  Constant5 : Constant  Value=P_AEB_Func_vehEBAObjSubjLatAccelActivationThresForPedCyc
  Logical Operator : Logic  Operator=OR  Inputs=3  AllPortsSameDT=off  OutDataTypeStr=boolean
  Logical Operator1 : Logic  AllPortsSameDT=off  OutDataTypeStr=boolean
  Logical Operator2 : Logic  AllPortsSameDT=off  OutDataTypeStr=boolean
  Logical Operator5 : Logic  Inputs=9  AllPortsSameDT=off  OutDataTypeStr=boolean
  PedCycObjCondSuppJudgeFlow : AEBSFunctionLib/PedCycObjCondSuppJudgeFlow  SystemSampleTime=-1  VariantActivationTime=update diagram  Latency=0  AutoFrameSizeCalculation=off
  Product : Product
  Relational Operator : RelationalOperator  Operator===  OutDataTypeStr=boolean  RndMeth=Simplest
  Unit Delay : UnitDelay  SampleTime=-1  HasFrameUpgradeWarning=on
  vehEBAObjRelActiveCondFulfilled : Outport
  wires:
  Compare To Constant10/1 -> Logical Operator5/9  "ObjFusQuality"
  ObjFusQuality/1 -> Compare To Constant10/1
  Compare To Constant8/1 -> Logical Operator5/3  "ObjFusionCameraConfirm"
  ObjectTypeCameraConfirmed/1 -> PedCycObjCondSuppJudgeFlow/1, Compare To Constant8/1
  ObjectType/1 -> PedCycObjCondSuppJudgeFlow/2
  subjCurvTraj/1 -> PedCycObjCondSuppJudgeFlow/3
  subjLatAccel/1 -> PedCycObjCondSuppJudgeFlow/4
  subjVel/1 -> PedCycObjCondSuppJudgeFlow/5
  vehConfigAEBCyc/1 -> PedCycObjCondSuppJudgeFlow/6
  vehConfigAEBPed/1 -> PedCycObjCondSuppJudgeFlow/7
  AEB_PedResp/1 -> PedCycObjCondSuppJudgeFlow/8
  Constant3/1 -> PedCycObjCondSuppJudgeFlow/9
  Constant4/1 -> PedCycObjCondSuppJudgeFlow/10
  Constant5/1 -> PedCycObjCondSuppJudgeFlow/11
  Compare To Constant7/1 -> Logical Operator5/2  "dxRelCondFulfilled"
  Compare To Constant9/1 -> Logical Operator5/8  "dxRelVarCondFulfilled"
  Logical Operator1/1 -> Logical Operator5/6  "objIDCondFulfilled"
  Logical Operator/1 -> Logical Operator5/1  "objMovingStateCondFulfilled"
  dxFrontBumper/1 -> Compare To Constant7/1
  PedCycObjCondSuppJudgeFlow/1 -> Compare To Constant12/1  "pedCycObjCondSuppFlg"
  Logical Operator2/1 -> Logical Operator5/7  "objQualityCondFulfilled"
  Compare To Constant3/1 -> Logical Operator5/5  "objMovingOrientationCondFulfilled"
  Logical Operator5/1 -> vehEBAObjRelActiveCondFulfilled/1  "vehEBAObjRelActiveCondFulfilled"
  Compare To Constant4/1 -> Logical Operator1/1
  Relational Operator/1 -> Logical Operator1/2
  dxVariance/1 -> Compare To Constant9/1
  Product/1 -> Compare To Constant5/1
  Compare To Constant5/1 -> Logical Operator2/2
  Compare To Constant6/1 -> Logical Operator2/1
  ObjectIsObstacleProb/1 -> Product/2
  ObjectExistProb/1 -> Product/1
  Unit Delay/1 -> Relational Operator/2
  ObjectID/1 -> Compare To Constant4/1, Relational Operator/1, Unit Delay/1, Compare To Constant6/1
  ObjectMovingOrientation/1 -> Compare To Constant3/1
  Compare To Constant1/1 -> Logical Operator/1
  Compare To Constant2/1 -> Logical Operator/2
  ObjectMovingState/1 -> Compare To Constant/1, Compare To Constant2/1, Compare To Constant1/1
  Compare To Constant12/1 -> Logical Operator5/4  "pedCycReleventCondFulfilled"
  Compare To Constant/1 -> Logical Operator/3

== system TosAeb/FcwAebJa_Step_sys/ALG_AEBSystem/ALG_AEBController/AEB_FuncCondCalcApp/LongFunctionCondCalc/vehEBASuppAborCondCalc/vehEBASuppCondCalc/vehEBASituationRelActiveCondCalc ==
  ObjectTypeCameraConfirmed : Inport
  dxFrontBumper : Inport  Port=2
  VehConfigIbooster : Inport  Port=3
  predictiveAccelAvoid : Inport  Port=4
  ObjectMovingState : Inport  Port=5
  subjVel : Inport  Port=6
  ESPmasterCylindBrakePress : Inport  Port=7
  BrakePedalStatus_G : Inport  Port=8
  subjLongAccelFromSensor : Inport  Port=9
  subjLongAccel : Inport  Port=10
  PreWarningFuncImpArb : Inport  Port=11
  overlapRatio : Inport  Port=12
  longCollisionSeverity : Inport  Port=13
  IB_BrkPedalAppld : Inport  Port=14
  ESPmasterCylindBrakePressDt : Subsystem
  brakeReleventSuppCond : Subsystem
  overlapThesCalc : Subsystem
  vehEBASituationRelActiveCondFulfilledCalc : Subsystem
  vehEBASituationRelActiveCondFulfilled : Outport
  wires:
  VehConfigIbooster/1 -> brakeReleventSuppCond/1(VehConfigIbooster)
  ESPmasterCylindBrakePressDt/1(ESPmasterCylindBrakePressDt) -> vehEBASituationRelActiveCondFulfilledCalc/7(ESPmasterCylindBrakePressDt)  "AEB_Func_vehEBA_MCBrkPressDt"
  IB_BrkPedalAppld/1 -> brakeReleventSuppCond/9(IB_BrkPedalAppld)
  longCollisionSeverity/1 -> vehEBASituationRelActiveCondFulfilledCalc/4(longCollisionSeverity)
  predictiveAccelAvoid/1 -> [predictiveAccelAvoid], brakeReleventSuppCond/2(predictiveAccelAvoid)
  [predictiveAccelAvoid] -> vehEBASituationRelActiveCondFulfilledCalc/1(predictiveAccelAvoid)
  PreWarningFuncImpArb/1 -> vehEBASituationRelActiveCondFulfilledCalc/2(PreWarningFuncImpArb)
  vehEBASituationRelActiveCondFulfilledCalc/1(vehEBASituationRelActiveCondFulfilled) -> vehEBASituationRelActiveCondFulfilled/1  "vehEBASituationRelActiveCondFulfilled"
  overlapThesCalc/1(overlapThes) -> vehEBASituationRelActiveCondFulfilledCalc/5(overlapThes)
  overlapRatio/1 -> vehEBASituationRelActiveCondFulfilledCalc/3(overlapRatio)
  brakeReleventSuppCond/1(brakeReleventSuppCond) -> vehEBASituationRelActiveCondFulfilledCalc/6(brkTriggerCondFulfilled)
  subjLongAccel/1 -> brakeReleventSuppCond/8(subjLongAccel)
  subjLongAccelFromSensor/1 -> brakeReleventSuppCond/7(subjLongAccelFromSensor)
  BrakePedalStatus_G/1 -> brakeReleventSuppCond/6(BrakePedalStatus_G)
  ESPmasterCylindBrakePress/1 -> ESPmasterCylindBrakePressDt/1(ESPmasterCylindBrakePress), brakeReleventSuppCond/5(ESPmasterCylindBrakePress)
  subjVel/1 -> brakeReleventSuppCond/4(subjVel)
  ObjectMovingState/1 -> brakeReleventSuppCond/3(ObjectMovingState)
  dxFrontBumper/1 -> overlapThesCalc/2(dxFrontBumper)
  ObjectTypeCameraConfirmed/1 -> overlapThesCalc/1(ObjectTypeCameraConfirmed)

== system TosAeb/FcwAebJa_Step_sys/ALG_AEBSystem/ALG_AEBController/AEB_FuncCondCalcApp/LongFunctionCondCalc/vehEBASuppAborCondCalc/vehEBASuppCondCalc/vehEBASuppCondFinalJudge ==
  vehEBAObjRelActiveCondFulfilled : Inport
  vehEBAVehRelActiveCondFulfilled : Inport  Port=2
  vehEBASituationRelActiveCondFulfilled : Inport  Port=3
  Compare To Constant : simulink/Logic and Bit Operations/Compare To Constant  relop===  const=FALSE  OutDataTypeStr=boolean
  Compare To Constant1 : simulink/Logic and Bit Operations/Compare To Constant  relop===  const=FALSE  OutDataTypeStr=boolean
  Compare To Constant2 : simulink/Logic and Bit Operations/Compare To Constant  relop===  const=FALSE  OutDataTypeStr=boolean
  Logical Operator : Logic  Operator=OR  Inputs=3  AllPortsSameDT=off  OutDataTypeStr=boolean
  vehEBASuppCondFulfilled : Outport
  wires:
  Compare To Constant2/1 -> Logical Operator/3
  Compare To Constant1/1 -> Logical Operator/2
  Compare To Constant/1 -> Logical Operator/1
  Logical Operator/1 -> vehEBASuppCondFulfilled/1  "vehEBASuppCondFulfilled"
  vehEBASituationRelActiveCondFulfilled/1 -> Compare To Constant2/1
  vehEBAObjRelActiveCondFulfilled/1 -> Compare To Constant/1
  vehEBAVehRelActiveCondFulfilled/1 -> Compare To Constant1/1

== system TosAeb/FcwAebJa_Step_sys/ALG_AEBSystem/ALG_AEBController/AEB_FuncCondCalcApp/LongFunctionCondCalc/vehEBASuppAborCondCalc/vehEBASuppCondCalc/vehEBAVehRelActiveCondCalc ==
  note: vehEBAVehRelActiveCondFulfilled
  subjVel : Inport
  ObjectMovingState : Inport  Port=2
  vehStateCalcEBASuppFlg : Inport  Port=3
  SteerWheelAngle : Inport  Port=4
  SteerWheelSpd : Inport  Port=5
  AccrPedlPosnDiagc : Inport  Port=6
  DriverCondJudge : Subsystem
  subjVelWork : Subsystem
  vehEBAVehRelActiveCondJudge : Subsystem
  vehEBAVehRelActiveCondFulfilled : Outport
  wires:
  subjVel/1 -> vehEBAVehRelActiveCondJudge/1(subjVel)
  vehEBAVehRelActiveCondJudge/1(vehEBAVehRelActiveCondFulfilled) -> vehEBAVehRelActiveCondFulfilled/1
  vehStateCalcEBASuppFlg/1 -> vehEBAVehRelActiveCondJudge/5(vehStateCalcEBASuppFlg)
  DriverCondJudge/1(driverSuppCondFlg) -> vehEBAVehRelActiveCondJudge/4(driverSuppCondFlg)
  ObjectMovingState/1 -> subjVelWork/1(ObjectMovingState)
  subjVelWork/1(subjVelWorkMax) -> vehEBAVehRelActiveCondJudge/2(subjVelWorkMax)
  subjVelWork/2(subjVelWorkMin) -> vehEBAVehRelActiveCondJudge/3(subjVelWorkMin)
  SteerWheelAngle/1 -> DriverCondJudge/1(SteerWheelAngle)
  SteerWheelSpd/1 -> DriverCondJudge/2(SteerWheelSpd)
  AccrPedlPosnDiagc/1 -> DriverCondJudge/3(AccrPedlPosnDiagc)

== system TosAeb/FcwAebJa_Step_sys/ALG_AEBSystem/ALG_AEBController/AEB_FuncCondCalcApp/LongFunctionCondCalc/vehLatentWarningSuppAborCondFulfilled/vehLatentWarningAborCondCalc/vehLatentWarningAborCondFinalJudge ==
  vehLatentWarningObjRelAborCondFulfilled : Inport
  vehLatentWarningVehRelAborCondFulfilled : Inport  Port=2
  vehLatentWarningSituationRelAborCondFulfilled : Inport  Port=3
  Compare To Constant : simulink/Logic and Bit Operations/Compare To Constant  relop===  const=TRUE  OutDataTypeStr=boolean
  Compare To Constant1 : simulink/Logic and Bit Operations/Compare To Constant  relop===  const=TRUE  OutDataTypeStr=boolean
  Compare To Constant2 : simulink/Logic and Bit Operations/Compare To Constant  relop===  const=TRUE  OutDataTypeStr=boolean
  Logical Operator : Logic  Operator=OR  Inputs=3  AllPortsSameDT=off  OutDataTypeStr=boolean
  vehLatentWarningAborCondFulfilled : Outport
  wires:
  vehLatentWarningVehRelAborCondFulfilled/1 -> Compare To Constant1/1
  vehLatentWarningObjRelAborCondFulfilled/1 -> Compare To Constant/1
  vehLatentWarningSituationRelAborCondFulfilled/1 -> Compare To Constant2/1
  Logical Operator/1 -> vehLatentWarningAborCondFulfilled/1  "vehPreWarningAborCondFulfilled"
  Compare To Constant/1 -> Logical Operator/1
  Compare To Constant1/1 -> Logical Operator/2
  Compare To Constant2/1 -> Logical Operator/3

== system TosAeb/FcwAebJa_Step_sys/ALG_AEBSystem/ALG_AEBController/AEB_FuncCondCalcApp/LongFunctionCondCalc/vehLatentWarningSuppAborCondFulfilled/vehLatentWarningAborCondCalc/vehLatentWarningObjRelActiveCondCalc ==
  ObjectID : Inport
  Compare To Constant4 : simulink/Logic and Bit Operations/Compare To Constant  relop===  const=CNobjInvalidID  OutDataTypeStr=boolean
  Logical Operator1 : Logic  Operator=OR  AllPortsSameDT=off  OutDataTypeStr=boolean
  Relational Operator : RelationalOperator  Operator=~=  OutDataTypeStr=boolean  RndMeth=Simplest
  Unit Delay : UnitDelay  SampleTime=-1  HasFrameUpgradeWarning=on
  vehLatentWarningObjRelActiveCondFulfilled : Outport
  wires:
  Logical Operator1/1 -> vehLatentWarningObjRelActiveCondFulfilled/1
  Compare To Constant4/1 -> Logical Operator1/1
  Relational Operator/1 -> Logical Operator1/2
  Unit Delay/1 -> Relational Operator/2
  ObjectID/1 -> Compare To Constant4/1, Relational Operator/1, Unit Delay/1
